simulink model "r_128w_1k_1a_16x_r430"  (Simulink 7.2)
settings: Solver=FixedStepDiscrete  SolverMode=SingleTasking  StartTime=0.0  StopTime=5000  RelTol=1e-3
undefined symbols (not defined in the file): Down, pfb_coeff_gen_calc, Up
libraries: xbsIndex_r4 x8495, casper_library_misc x233, casper_library_delays x150, xps_library x70, simulink x28, dspsigops x12, casper_library_flow_control x8, casper_library_multipliers x8, xbsReplacements_r4 x4, casper_library_ffts_twiddle x2
(part 46 of 112)

== system r_128w_1k_1a_16x_r430/fft_wideband_real0/fft_direct/butterfly2_0/twiddle_general_4mult ==
  a : Inport
  b : Inport  Port=2
  sync : Inport  Port=3
  AddSub : xbsIndex_r4/AddSub  mode=Subtraction  use_carryin=off  use_carryout=off  en=off  latency=add_latency  precision=Full  arith_type=Unsigned  n_bits=16  bin_pt=14  quantization=Truncate  overflow=Wrap  dbl_ovrd=off  use_behavioral_HDL=on  hw_selection=Fabric  pipelined=off  block_type=addsub
  AddSub1 : xbsIndex_r4/AddSub  mode=Addition  use_carryin=off  use_carryout=off  en=off  latency=add_latency  precision=Full  arith_type=Unsigned  n_bits=16  bin_pt=14  quantization=Truncate  overflow=Wrap  dbl_ovrd=off  use_behavioral_HDL=on  hw_selection=Fabric  pipelined=off  block_type=addsub
  c_to_ri1 : casper_library_misc/c_to_ri  SystemSampleTime=-1  n_bits=input_bit_width  bin_pt=input_bit_width-1
  c_to_ri2 : casper_library_misc/c_to_ri  SystemSampleTime=-1  n_bits=coeff_bit_width  bin_pt=coeff_bit_width-2
      settings: AttributesFormatString=18_16 r/i
  c_to_ri3 : casper_library_misc/c_to_ri  SystemSampleTime=-1  n_bits=input_bit_width  bin_pt=input_bit_width-1
  coeff_gen : Subsystem  AncestorBlock=casper_library_ffts_twiddle_coeff_gen/coeff_gen  mask(coeff_gen){Coeffs=[1+0i       0.99999529380957619117964441102231-0.0030679567629659761432425746363606i       0.99998117528260110908888691483298-0.0061358846491544752690949771078976i       0.99995764455196389786095778617891-0.0092037547820598194364682953505508i        0.99992470183914450299056397852837-0.012271538285719925387429185548172i        0.99988234745421256111086449891445-0.015339206284988100154054180279672i        0.99983058179582340319058175737155-0.018406729905804820185410974886508i        0.99976940535121527897644000404398-0.021474080275469507872454499874948i        0.99969881869620424996725205346593-0.024541228522912288123603019585062i        0.99961882249517863829879615877871-0.027608145778965739736143802929291i        0.99952941750109314256178549840115-0.030674803176636625950957082409332i         0.99943060455546173237451057502767-0.03374117185137757990442963773603i        0.99932238458834954375475945198559-0.036807222941358831713731802892653i        0.99920475861836388631331828946713-0.039872927587739810662004202868047i        0.99907772775264536146977434327709-0.042938256934940820241930481415693i        0.99894129318685687124457217578311-0.046003182130914622993689278018792i        0.99879545620517240500646494183457-0.049067674327418014934565348994511i        0.99864021818026527110845336210332-0.052131704680283323660727745618715i        0.99847558057329477421149022120517-0.055195244349689941143477511786841i        0.99830154493389289260818486582139-0.058258264500435752442086823066347i        0.99811811290014917918966830256977-0.061320736302208578294425933563616i        0.99792528619859599547936568342266-0.064382630929857465051924236831837i           0.9977230666441916362430220033275-0.0674439195636640509423642697584i        0.99751145614030345409872779782745-0.070504573389613856004842773472774i        0.99729045667869020697082760307239-0.073564563599667426307959772202594i        0.99706007033948296225389640312642-0.076623861392031492045795459944202i        0.99682029929116566790980868972838-0.079682437971430125633887087133189i         0.9965711457905548353863878219272-0.082740264549375691638388730098086i          0.996312612182778001290728298045-0.085797312344439893849745715215249i        0.99604470090125196701791310260887-0.088853552582524600311764118032443i        0.99576741446765981713440396561055-0.091908956497132723861831493650243i        0.99548075549192693856070945912506-0.094963495329638991648835144587792i        0.99518472667219692873175063141389-0.098017140329560603628777926132898i         0.99487933079480561637808477826184-0.10106986275482782167145501261984i         0.99456457073425541537403660186101-0.10412163387205458642270627933613i         0.99424044945318790222898996944423-0.10717242495680884273312472032558i         0.99390697000235606051177228437155-0.11022220729388305937579417559391i           0.99356413552059530402971176954452-0.113270952177564346308180631695i         0.99321194923479450000769475082052-0.11631863091190475234526502390509i          0.9928504144598651048880810776609-0.11936521481099135466585892118019i         0.99247953459870996706371215623221-0.12241067519921619566325432515441i         0.99209931314219179654401159496047-0.12545498341154623367366127695277i         0.99170975366909952519733906228794-0.12849811079379316880455519367388i         0.99131085984611544414946138203959-0.13154002870288311610735831891361i         0.99090263542778000971367191596073-0.13458070850712616772781871077314i          0.9904850842564570934101197963173-0.13762012158648603832311607675365i          0.99005821026229712256139237069874-0.1406582393328492108786065273307i          0.98962201746320088702191242191475-0.1436950331502944711026259483333i         0.98917650996478101443898367506335-0.14673047445536174793190298260015i         0.98872169196032377858074369214592-0.14976453467732150914848432421422i         0.98825756773074946437418475397862-0.15279718525844343535169400638551i ...(+36410 chars)], coeff_bit_width=coeff_bit_width, StepPeriod=0, bram_latency=bram_latency, coeffs_bram=on}
      mask prompts: Coeffs "Coefficients: (0 to 2^(FFTSize-1)-1)"; coeff_bit_width "Coefficient Bit Width:"; StepPeriod "Step Period: (2^?)"; bram_latency "Latency:"; coeffs_bram "Store coefficients in BRAM"
      mask help: Generates a sequence of complex coefficients
      mask init:
      | coeff_gen_init( gcb, ...
      |     'Coeffs', Coeffs, ...
      |     'coeff_bit_width', coeff_bit_width, ...
      |     'StepPeriod', StepPeriod, ...
      |     'bram_latency', bram_latency, ...
      |     'coeffs_bram', coeffs_bram);
      settings: AttributesFormatString=512 @ (18,16)
      mask CallbackString:
      | Coeffs = eval(get_param(gcb, 'Coeffs'));
      | if( length(Coeffs) == 1 ),
      |     set_param(gcb, 'MaskVisibilities',{'on','on','off','off','off'});
      | else
      |     set_param(gcb, 'MaskVisibilities',{'on','on','on','on','on'});
      | end||||
  convert0 : xbsIndex_r4/Convert  arith_type="Signed  (2's comp)"  n_bits=input_bit_width+4  bin_pt=input_bit_width+1  quantization="Round  (unbiased: Even Values)"  overflow=Wrap  latency=conv_latency  dbl_ovrd=off  pipeline=on  block_type=convert
      settings: block_version=11.4
  convert1 : xbsIndex_r4/Convert  arith_type="Signed  (2's comp)"  n_bits=input_bit_width+4  bin_pt=input_bit_width+1  quantization="Round  (unbiased: Even Values)"  overflow=Wrap  latency=conv_latency  dbl_ovrd=off  pipeline=on  block_type=convert
      settings: block_version=11.4
  delay0 : xbsIndex_r4/Delay  en=off  latency=mult_latency + add_latency + bram_latency + conv_latency  dbl_ovrd=off  reg_retiming=off  block_type=delay
      settings: block_version=11.4
  delay1 : xbsIndex_r4/Delay  en=off  latency=bram_latency  dbl_ovrd=off  reg_retiming=on  block_type=delay
      settings: block_version=11.4
  delay2 : xbsIndex_r4/Delay  en=off  latency=mult_latency + add_latency + bram_latency + conv_latency  dbl_ovrd=off  reg_retiming=off  block_type=delay
      settings: block_version=11.4
  mult{,1..3} (4 alike) : xbsIndex_r4/Mult  precision=Full  arith_type="Signed  (2's comp)"  n_bits=16  bin_pt=14  quantization=Truncate  overflow=Wrap  en=off  latency=mult_latency  dbl_ovrd=off  use_behavioral_HDL=on  opt=Speed  use_embedded=off  optimum_pipeline=off  pipeline=on  use_rpm=on  placement_style=Triangular  block_type=mult
      settings: block_version=11.4
  a_re : Outport
  a_im : Outport  Port=2
  bw_re : Outport  Port=3
  bw_im : Outport  Port=4
  sync_out : Outport  Port=5
  wires:
  a/1 -> delay0/1
  delay0/1 -> c_to_ri1/1
  c_to_ri1/1 -> a_re/1
  c_to_ri1/2 -> a_im/1
  sync/1 -> delay2/1, coeff_gen/1(rst)
  delay2/1 -> sync_out/1
  coeff_gen/1(w) -> c_to_ri2/1
  b/1 -> delay1/1
  delay1/1 -> c_to_ri3/1
  c_to_ri3/1 -> mult/1, mult3/1
  c_to_ri2/1 -> mult/2, mult1/2
  c_to_ri3/2 -> mult1/1, mult2/1
  c_to_ri2/2 -> mult2/2, mult3/2
  mult/1 -> AddSub/1
  mult1/1 -> AddSub1/1
  mult2/1 -> AddSub/2
  mult3/1 -> AddSub1/2
  AddSub/1 -> convert0/1
  convert0/1 -> bw_re/1
  AddSub1/1 -> convert1/1
  convert1/1 -> bw_im/1
